simulink model "mb1k-v159"  (R2013a)
settings: Solver=VariableStepDiscrete  SolverMode=SingleTasking  StartTime=0.0  StopTime=10^4  FixedStep=1  MaxStep=1  RelTol=1e-3
undefined symbols (not defined in the file): pfb_coeff_gen_calc, sfp
libraries: xbsIndex_r4 x25251, casper_library_misc x140, casper_library_delays x74, simulink x34, dspsigops x24, xbsReplacements_r4 x20, fast_library x16, xps_library x13, casper_library_sources x2
(part 3 of 256)

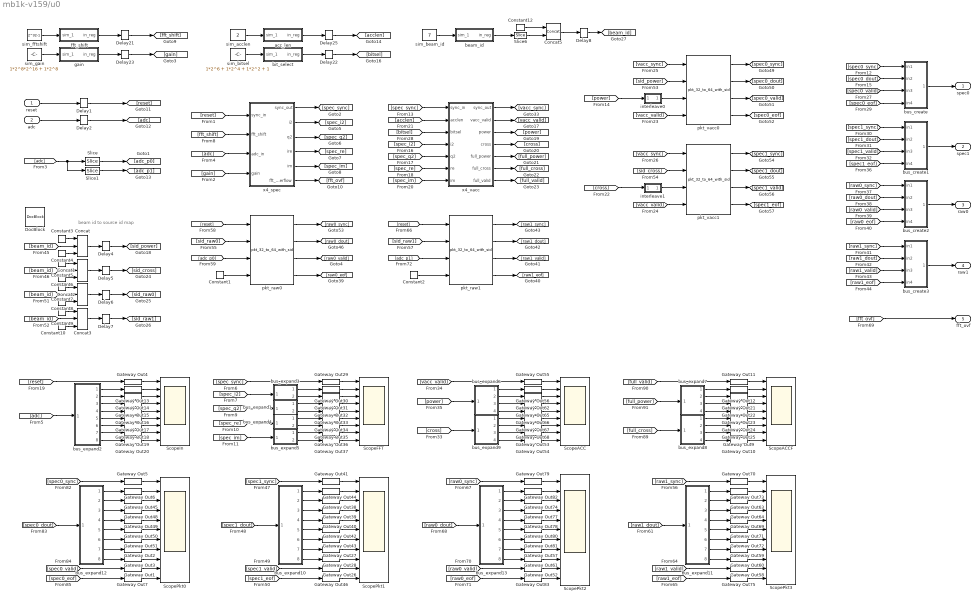
== system mb1k-v159/u0 ==
  note: beam id to source id map
  reset : Inport
  adc : Inport  Port=2
  Concat{,1..3} (4 alike) : xbsIndex_r4/Concat  num_inputs=3  dbl_ovrd=off  block_type=concat
  Concat5 : xbsIndex_r4/Concat  num_inputs=2  dbl_ovrd=off  block_type=concat
  Constant{1..2,6..7,9..10} (6 alike) : xbsIndex_r4/Constant  const=1  gui_display_data_type=Boolean  arith_type=Boolean  n_bits=16  bin_pt=14  preci_type=Single  exp_width=8  frac_width=24  explicit_period=on  period=1  equ=P=C  opselect=C  inp2=PCIN>>17  opr=+  inp1=P  carry=CIN  dbl_ovrd=off  block_type=constant
  Constant12 : xbsIndex_r4/Constant  const=1  gui_display_data_type=Fixed-point  arith_type=Unsigned  n_bits=1  bin_pt=0  preci_type=Single  exp_width=8  frac_width=24  explicit_period=on  period=1  equ=P=C  opselect=C  inp2=PCIN>>17  opr=+  inp1=P  carry=CIN  dbl_ovrd=off  block_type=constant
  Constant{3..5,8} (4 alike) : xbsIndex_r4/Constant  const=0  gui_display_data_type=Boolean  arith_type=Boolean  n_bits=16  bin_pt=14  preci_type=Single  exp_width=8  frac_width=24  explicit_period=on  period=1  equ=P=C  opselect=C  inp2=PCIN>>17  opr=+  inp1=P  carry=CIN  dbl_ovrd=off  block_type=constant
  Delay{1..2,4..8,21..23,25} (11 alike) : xbsIndex_r4/Delay  rst=off  infoeditControl=Selection of Reset will increase slice count due to use of real FFs and instead of SRLs  en=off  latency=1  dbl_ovrd=off  reg_retiming=off  block_type=delay
  DocBlock : simulink/Model-Wide Utilities/DocBlock  DocumentType=Text
      content=|
      | Highest byte of 64-bit little endian packet serial number to represent source id:
      |
      | bit 7:    0 - power spectra, 1 - pure ADC sample
      | bit 6:    0 - single beam, 1 - multi-beam
      | bit 5-1:  5 bits, 32 values for beam id.
      |           multi-beam: 1-19 (I reserved number 0)
      |           single-beam: TBD
      | bit 0:    ADC data: polarization
      |           spectra: 0 - power term, 1 - cross term
  Gateway Out{1..7,9..46,48..75,77..83} (80 alike) : xbsIndex_r4/Gateway Out  inherit_from_input=off  hdl_port=on  timing_constraint=None  locs_specified=off  LOCs={}  UseAsDAC=off  DACChannel='1'  block_type=gatewayout
  ScopeACC : Scope  NumInputPorts=9
      settings: YMin=-5~-5~-5~-5~-5~-5~-5~-5~-5  YMax=5~5~5~5~5~5~5~5~5  SaveName=u0ScopeACC
  ScopeACCF : Scope  NumInputPorts=9
      settings: YMin=-5~-5~-5~-5~-5~-5~-5~-5~-5  YMax=5~5~5~5~5~5~5~5~5  SaveName=u0ScopeACCF
  ScopeFFT : Scope  NumInputPorts=9
      settings: YMin=-5~-5~-5~-5~-5~-5~-5~-5~-5  YMax=5~5~5~5~5~5~5~5~5  SaveName=u0ScopeFFT
  ScopeIn : Scope  NumInputPorts=9
      settings: YMin=-5~-0.1~-5~-5~-5~-5~-5~-5~-5  YMax=5~0.1~5~5~5~5~5~5~5  SaveName=u0ScopeIn
  ScopePkt{0..3} (4 alike) : Scope  NumInputPorts=11
      ScopePkt0 settings: YMin=-5~-5~-5~-5~-5~-5~-5~-5~-5~-5~-5  YMax=5~5~5~5~5~5~5~5~5~5~5  SaveName=u0ScopePkt0
      ScopePkt1 settings: YMin=-5~-5~-5~-5~-5~-5~-5~-5~-5~-5~-5  YMax=5~5~5~5~5~5~5~5~5~5~5  SaveName=u0ScopePkt1
      ScopePkt2 settings: YMin=-5~-5~-5~-5~-5~-5~-5~-5~-5~-5~-5  YMax=5~5~5~5~5~5~5~5~5~5~5  SaveName=u0ScopePkt2
      ScopePkt3 settings: YMin=-5~-5~-5~-5~-5~-5~-5~-5~-5~-5~-5  YMax=5~5~5~5~5~5~5~5~5~5~5  SaveName=u0ScopePkt3
  Slice : xbsIndex_r4/Slice  nbits=32  boolean_output=off  mode=Upper Bit Location + Width  bit1=0  base1=MSB of Input  bit0=0  base0=LSB of Input  dbl_ovrd=off  block_type=slice
  Slice1 : xbsIndex_r4/Slice  nbits=32  boolean_output=off  mode=Lower Bit Location + Width  bit1=0  base1=MSB of Input  bit0=0  base0=LSB of Input  dbl_ovrd=off  block_type=slice
  Slice6 : xbsIndex_r4/Slice  nbits=5  boolean_output=off  mode=Lower Bit Location + Width  bit1=0  base1=MSB of Input  bit0=0  base0=LSB of Input  dbl_ovrd=off  block_type=slice
  acc_len : Subsystem  Tag=xps:sw_reg  AncestorBlock=xps_library/software register  mask(swreg){io_dir=From Processor, io_delay=0, sample_period=1, names=reg, bitwidths=20, bin_pts=0, arith_types=0, sim_port=on, show_format=off}
      mask prompts: io_dir "I/O direction"; io_delay "I/O delay"; sample_period "Sample period"; names "Bitfield names [msb...lsb]"; bitwidths "Bitfield widths"; bin_pts "Bitfield binary pts"; arith_types "Bitfield types, ufix=0, fix=1, bool=2"; sim_port "Provide sim input/output?"; show_format "Print format string?"
      mask help: A 32-bit software-accessible register. Can be divided into bitfields of varying widths and types using the fields in the block mask.
      mask init:
      | swreg_init(gcb)
      settings: AttributesFormatString=1input
      mask TypeOptions io_dir: To Processor | From Processor
      mask Callback io_dir:
      | mask_enables = get_param(gcb, 'MaskEnables');
      | mask_names = get_param(gcb, 'MaskNames');
      | if strcmp(get_param(gcb, 'io_dir'), 'To Processor'),
      |     mask_enables{ismember(mask_names, 'sample_period')} = 'off';
      | else
      |     mask_enables{ismember(mask_names, 'sample_period')} = 'on';
      | end
      | set_param(gcb, 'MaskEnables', mask_enables);
  beam_id : Subsystem  Tag=xps:sw_reg  AncestorBlock=xps_library/software register  mask(swreg){io_dir=From Processor, io_delay=0, sample_period=1, names=reg, bitwidths=5, bin_pts=0, arith_types=0, sim_port=on, show_format=off}
      mask prompts: io_dir "I/O direction"; io_delay "I/O delay"; sample_period "Sample period"; names "Bitfield names [msb...lsb]"; bitwidths "Bitfield widths"; bin_pts "Bitfield binary pts"; arith_types "Bitfield types, ufix=0, fix=1, bool=2"; sim_port "Provide sim input/output?"; show_format "Print format string?"
      mask help: A 32-bit software-accessible register. Can be divided into bitfields of varying widths and types using the fields in the block mask.
      mask init:
      | swreg_init(gcb)
      settings: AttributesFormatString=1input
      mask TypeOptions io_dir: To Processor | From Processor
      mask Callback io_dir:
      | mask_enables = get_param(gcb, 'MaskEnables');
      | mask_names = get_param(gcb, 'MaskNames');
      | if strcmp(get_param(gcb, 'io_dir'), 'To Processor'),
      |     mask_enables{ismember(mask_names, 'sample_period')} = 'off';
      | else
      |     mask_enables{ismember(mask_names, 'sample_period')} = 'on';
      | end
      | set_param(gcb, 'MaskEnables', mask_enables);
  bit_select : Subsystem  Tag=xps:sw_reg  AncestorBlock=xps_library/software register  mask(swreg){io_dir=From Processor, io_delay=0, sample_period=1, names=reg, bitwidths=8, bin_pts=0, arith_types=0, sim_port=on, show_format=off}
      mask prompts: io_dir "I/O direction"; io_delay "I/O delay"; sample_period "Sample period"; names "Bitfield names [msb...lsb]"; bitwidths "Bitfield widths"; bin_pts "Bitfield binary pts"; arith_types "Bitfield types, ufix=0, fix=1, bool=2"; sim_port "Provide sim input/output?"; show_format "Print format string?"
      mask help: A 32-bit software-accessible register. Can be divided into bitfields of varying widths and types using the fields in the block mask.
      mask init:
      | swreg_init(gcb)
      settings: AttributesFormatString=1input
      mask TypeOptions io_dir: To Processor | From Processor
      mask Callback io_dir:
      | mask_enables = get_param(gcb, 'MaskEnables');
      | mask_names = get_param(gcb, 'MaskNames');
      | if strcmp(get_param(gcb, 'io_dir'), 'To Processor'),
      |     mask_enables{ismember(mask_names, 'sample_period')} = 'off';
      | else
      |     mask_enables{ismember(mask_names, 'sample_period')} = 'on';
      | end
      | set_param(gcb, 'MaskEnables', mask_enables);
  bus_create : Subsystem  AncestorBlock=casper_library_flow_control/bus_create  mask(bus_create){inputNum=4}
      mask prompts: inputNum "Number of inputs:"
      mask init:
      | bus_create_init(gcb,...
      |     'inputNum', inputNum);
  bus_create1 : Subsystem  AncestorBlock=casper_library_flow_control/bus_create  mask(bus_create){inputNum=4}
      mask prompts: inputNum "Number of inputs:"
      mask init:
      | bus_create_init(gcb,...
      |     'inputNum', inputNum);
  bus_create2 : Subsystem  AncestorBlock=casper_library_flow_control/bus_create  mask(bus_create){inputNum=4}
      mask prompts: inputNum "Number of inputs:"
      mask init:
      | bus_create_init(gcb,...
      |     'inputNum', inputNum);
  bus_create3 : Subsystem  AncestorBlock=casper_library_flow_control/bus_create  mask(bus_create){inputNum=4}
      mask prompts: inputNum "Number of inputs:"
      mask init:
      | bus_create_init(gcb,...
      |     'inputNum', inputNum);
  bus_expand1 : Subsystem  AncestorBlock=casper_library_flow_control/bus_expand  mask(bus_expand){mode=divisions of equal size, outputNum=2, outputWidth=36, outputBinaryPt=34, outputArithmeticType=0, show_format=off, outputToWorkspace=off, variablePrefix=out, outputToModelAsWell=on}
      mask prompts: outputNum "Number of outputs:"; outputWidth "Output width:"; outputBinaryPt "Output binary point position:"; outputArithmeticType "Output arithmetic type (ufix=0, fix=1, bool=2):"; show_format "Print format string?"; outputToWorkspace "Output to workspace?"; variablePrefix "Variable name prefix:"; outputToModelAsWell "Output to model as well?"
      mask help:
      | Expands a 'bus' made using a bus_create or Xilinx
      | concat block.
      | The input will be divided into the specified number
      | of outputs with the specified arithmetic types.
      | Divisions are made from most significant bit.
      mask init: same as mb1k-v159/bus_expand
      settings: AttributesFormatString=2 outputs
      mask TypeOptions mode: divisions of equal size | divisions of arbitrary size
      mask Callback mode: bus_expand_callback();
      mask Callback outputWidth: bus_expand_callback();
      mask Callback outputToWorkspace: bus_expand_callback();
  bus_expand10 : Subsystem  AncestorBlock=casper_library_flow_control/bus_expand  mask(bus_expand){mode=divisions of equal size, outputNum=8, outputWidth=8, outputBinaryPt=0, outputArithmeticType=0, show_format=off, outputToWorkspace=off, variablePrefix=out, outputToModelAsWell=on}
      mask prompts: outputNum "Number of outputs:"; outputWidth "Output width:"; outputBinaryPt "Output binary point position:"; outputArithmeticType "Output arithmetic type (ufix=0, fix=1, bool=2):"; show_format "Print format string?"; outputToWorkspace "Output to workspace?"; variablePrefix "Variable name prefix:"; outputToModelAsWell "Output to model as well?"
      mask help:
      | Expands a 'bus' made using a bus_create or Xilinx
      | concat block.
      | The input will be divided into the specified number
      | of outputs with the specified arithmetic types.
      | Divisions are made from most significant bit.
      mask init: same as mb1k-v159/bus_expand
      settings: AttributesFormatString=8 outputs
      mask TypeOptions mode: divisions of equal size | divisions of arbitrary size
      mask Callback mode: bus_expand_callback();
      mask Callback outputWidth: bus_expand_callback();
      mask Callback outputToWorkspace: bus_expand_callback();
  bus_expand11 : Subsystem  AncestorBlock=casper_library_flow_control/bus_expand  mask(bus_expand){mode=divisions of equal size, outputNum=8, outputWidth=8, outputBinaryPt=0, outputArithmeticType=0, show_format=off, outputToWorkspace=off, variablePrefix=out, outputToModelAsWell=on}
      mask prompts: outputNum "Number of outputs:"; outputWidth "Output width:"; outputBinaryPt "Output binary point position:"; outputArithmeticType "Output arithmetic type (ufix=0, fix=1, bool=2):"; show_format "Print format string?"; outputToWorkspace "Output to workspace?"; variablePrefix "Variable name prefix:"; outputToModelAsWell "Output to model as well?"
      mask help:
      | Expands a 'bus' made using a bus_create or Xilinx
      | concat block.
      | The input will be divided into the specified number
      | of outputs with the specified arithmetic types.
      | Divisions are made from most significant bit.
      mask init: same as mb1k-v159/bus_expand
      settings: AttributesFormatString=8 outputs
      mask TypeOptions mode: divisions of equal size | divisions of arbitrary size
      mask Callback mode: bus_expand_callback();
      mask Callback outputWidth: bus_expand_callback();
      mask Callback outputToWorkspace: bus_expand_callback();
  bus_expand12 : Subsystem  AncestorBlock=casper_library_flow_control/bus_expand  mask(bus_expand){mode=divisions of equal size, outputNum=8, outputWidth=8, outputBinaryPt=0, outputArithmeticType=0, show_format=off, outputToWorkspace=off, variablePrefix=out, outputToModelAsWell=on}
      mask prompts: outputNum "Number of outputs:"; outputWidth "Output width:"; outputBinaryPt "Output binary point position:"; outputArithmeticType "Output arithmetic type (ufix=0, fix=1, bool=2):"; show_format "Print format string?"; outputToWorkspace "Output to workspace?"; variablePrefix "Variable name prefix:"; outputToModelAsWell "Output to model as well?"
      mask help:
      | Expands a 'bus' made using a bus_create or Xilinx
      | concat block.
      | The input will be divided into the specified number
      | of outputs with the specified arithmetic types.
      | Divisions are made from most significant bit.
      mask init: same as mb1k-v159/bus_expand
      settings: AttributesFormatString=8 outputs
      mask TypeOptions mode: divisions of equal size | divisions of arbitrary size
      mask Callback mode: bus_expand_callback();
      mask Callback outputWidth: bus_expand_callback();
      mask Callback outputToWorkspace: bus_expand_callback();
  bus_expand13 : Subsystem  AncestorBlock=casper_library_flow_control/bus_expand  mask(bus_expand){mode=divisions of equal size, outputNum=8, outputWidth=8, outputBinaryPt=0, outputArithmeticType=0, show_format=off, outputToWorkspace=off, variablePrefix=out, outputToModelAsWell=on}
      mask prompts: outputNum "Number of outputs:"; outputWidth "Output width:"; outputBinaryPt "Output binary point position:"; outputArithmeticType "Output arithmetic type (ufix=0, fix=1, bool=2):"; show_format "Print format string?"; outputToWorkspace "Output to workspace?"; variablePrefix "Variable name prefix:"; outputToModelAsWell "Output to model as well?"
      mask help:
      | Expands a 'bus' made using a bus_create or Xilinx
      | concat block.
      | The input will be divided into the specified number
      | of outputs with the specified arithmetic types.
      | Divisions are made from most significant bit.
      mask init: same as mb1k-v159/bus_expand
      settings: AttributesFormatString=8 outputs
      mask TypeOptions mode: divisions of equal size | divisions of arbitrary size
      mask Callback mode: bus_expand_callback();
      mask Callback outputWidth: bus_expand_callback();
      mask Callback outputToWorkspace: bus_expand_callback();
  bus_expand2 : Subsystem  AncestorBlock=casper_library_flow_control/bus_expand  mask(bus_expand){mode=divisions of equal size, outputNum=8, outputWidth=8, outputBinaryPt=7, outputArithmeticType=1, show_format=off, outputToWorkspace=off, variablePrefix=out, outputToModelAsWell=on}
      mask prompts: outputNum "Number of outputs:"; outputWidth "Output width:"; outputBinaryPt "Output binary point position:"; outputArithmeticType "Output arithmetic type (ufix=0, fix=1, bool=2):"; show_format "Print format string?"; outputToWorkspace "Output to workspace?"; variablePrefix "Variable name prefix:"; outputToModelAsWell "Output to model as well?"
      mask help:
      | Expands a 'bus' made using a bus_create or Xilinx
      | concat block.
      | The input will be divided into the specified number
      | of outputs with the specified arithmetic types.
      | Divisions are made from most significant bit.
      mask init: same as mb1k-v159/bus_expand
      settings: AttributesFormatString=8 outputs
      mask TypeOptions mode: divisions of equal size | divisions of arbitrary size
      mask Callback mode: bus_expand_callback();
      mask Callback outputWidth: bus_expand_callback();
      mask Callback outputToWorkspace: bus_expand_callback();
  bus_expand3 : Subsystem  AncestorBlock=casper_library_flow_control/bus_expand  mask(bus_expand){mode=divisions of equal size, outputNum=2, outputWidth=36, outputBinaryPt=34, outputArithmeticType=0, show_format=off, outputToWorkspace=off, variablePrefix=out, outputToModelAsWell=on}
      mask prompts: outputNum "Number of outputs:"; outputWidth "Output width:"; outputBinaryPt "Output binary point position:"; outputArithmeticType "Output arithmetic type (ufix=0, fix=1, bool=2):"; show_format "Print format string?"; outputToWorkspace "Output to workspace?"; variablePrefix "Variable name prefix:"; outputToModelAsWell "Output to model as well?"
      mask help:
      | Expands a 'bus' made using a bus_create or Xilinx
      | concat block.
      | The input will be divided into the specified number
      | of outputs with the specified arithmetic types.
      | Divisions are made from most significant bit.
      mask init: same as mb1k-v159/bus_expand
      settings: AttributesFormatString=2 outputs
      mask TypeOptions mode: divisions of equal size | divisions of arbitrary size
      mask Callback mode: bus_expand_callback();
      mask Callback outputWidth: bus_expand_callback();
      mask Callback outputToWorkspace: bus_expand_callback();
  bus_expand4 : Subsystem  AncestorBlock=casper_library_flow_control/bus_expand  mask(bus_expand){mode=divisions of equal size, outputNum=2, outputWidth=36, outputBinaryPt=34, outputArithmeticType=1, show_format=off, outputToWorkspace=off, variablePrefix=out, outputToModelAsWell=on}
      mask prompts: outputNum "Number of outputs:"; outputWidth "Output width:"; outputBinaryPt "Output binary point position:"; outputArithmeticType "Output arithmetic type (ufix=0, fix=1, bool=2):"; show_format "Print format string?"; outputToWorkspace "Output to workspace?"; variablePrefix "Variable name prefix:"; outputToModelAsWell "Output to model as well?"
      mask help:
      | Expands a 'bus' made using a bus_create or Xilinx
      | concat block.
      | The input will be divided into the specified number
      | of outputs with the specified arithmetic types.
      | Divisions are made from most significant bit.
      mask init: same as mb1k-v159/bus_expand
      settings: AttributesFormatString=2 outputs
      mask TypeOptions mode: divisions of equal size | divisions of arbitrary size
      mask Callback mode: bus_expand_callback();
      mask Callback outputWidth: bus_expand_callback();
      mask Callback outputToWorkspace: bus_expand_callback();
  bus_expand5 : Subsystem  AncestorBlock=casper_library_flow_control/bus_expand  mask(bus_expand){mode=divisions of equal size, outputNum=2, outputWidth=36, outputBinaryPt=34, outputArithmeticType=1, show_format=off, outputToWorkspace=off, variablePrefix=out, outputToModelAsWell=on}
      mask prompts: outputNum "Number of outputs:"; outputWidth "Output width:"; outputBinaryPt "Output binary point position:"; outputArithmeticType "Output arithmetic type (ufix=0, fix=1, bool=2):"; show_format "Print format string?"; outputToWorkspace "Output to workspace?"; variablePrefix "Variable name prefix:"; outputToModelAsWell "Output to model as well?"
      mask help:
      | Expands a 'bus' made using a bus_create or Xilinx
      | concat block.
      | The input will be divided into the specified number
      | of outputs with the specified arithmetic types.
      | Divisions are made from most significant bit.
      mask init: same as mb1k-v159/bus_expand
      settings: AttributesFormatString=2 outputs
      mask TypeOptions mode: divisions of equal size | divisions of arbitrary size
      mask Callback mode: bus_expand_callback();
      mask Callback outputWidth: bus_expand_callback();
      mask Callback outputToWorkspace: bus_expand_callback();
  bus_expand6 : Subsystem  AncestorBlock=casper_library_flow_control/bus_expand  mask(bus_expand){mode=divisions of equal size, outputNum=4, outputWidth=8, outputBinaryPt=0, outputArithmeticType=0, show_format=off, outputToWorkspace=off, variablePrefix=out, outputToModelAsWell=on}
      mask prompts: outputNum "Number of outputs:"; outputWidth "Output width:"; outputBinaryPt "Output binary point position:"; outputArithmeticType "Output arithmetic type (ufix=0, fix=1, bool=2):"; show_format "Print format string?"; outputToWorkspace "Output to workspace?"; variablePrefix "Variable name prefix:"; outputToModelAsWell "Output to model as well?"
      mask help:
      | Expands a 'bus' made using a bus_create or Xilinx
      | concat block.
      | The input will be divided into the specified number
      | of outputs with the specified arithmetic types.
      | Divisions are made from most significant bit.
      mask init: same as mb1k-v159/bus_expand
      settings: AttributesFormatString=4 outputs
      mask TypeOptions mode: divisions of equal size | divisions of arbitrary size
      mask Callback mode: bus_expand_callback();
      mask Callback outputWidth: bus_expand_callback();
      mask Callback outputToWorkspace: bus_expand_callback();
  bus_expand7 : Subsystem  AncestorBlock=casper_library_flow_control/bus_expand  mask(bus_expand){mode=divisions of equal size, outputNum=4, outputWidth=32, outputBinaryPt=0, outputArithmeticType=0, show_format=off, outputToWorkspace=off, variablePrefix=out, outputToModelAsWell=on}
      mask prompts: outputNum "Number of outputs:"; outputWidth "Output width:"; outputBinaryPt "Output binary point position:"; outputArithmeticType "Output arithmetic type (ufix=0, fix=1, bool=2):"; show_format "Print format string?"; outputToWorkspace "Output to workspace?"; variablePrefix "Variable name prefix:"; outputToModelAsWell "Output to model as well?"
      mask help:
      | Expands a 'bus' made using a bus_create or Xilinx
      | concat block.
      | The input will be divided into the specified number
      | of outputs with the specified arithmetic types.
      | Divisions are made from most significant bit.
      mask init: same as mb1k-v159/bus_expand
      settings: AttributesFormatString=4 outputs
      mask TypeOptions mode: divisions of equal size | divisions of arbitrary size
      mask Callback mode: bus_expand_callback();
      mask Callback outputWidth: bus_expand_callback();
      mask Callback outputToWorkspace: bus_expand_callback();
  bus_expand8 : Subsystem  AncestorBlock=casper_library_flow_control/bus_expand  mask(bus_expand){mode=divisions of equal size, outputNum=4, outputWidth=32, outputBinaryPt=0, outputArithmeticType=1, show_format=off, outputToWorkspace=off, variablePrefix=out, outputToModelAsWell=on}
      mask prompts: outputNum "Number of outputs:"; outputWidth "Output width:"; outputBinaryPt "Output binary point position:"; outputArithmeticType "Output arithmetic type (ufix=0, fix=1, bool=2):"; show_format "Print format string?"; outputToWorkspace "Output to workspace?"; variablePrefix "Variable name prefix:"; outputToModelAsWell "Output to model as well?"
      mask help:
      | Expands a 'bus' made using a bus_create or Xilinx
      | concat block.
      | The input will be divided into the specified number
      | of outputs with the specified arithmetic types.
      | Divisions are made from most significant bit.
      mask init: same as mb1k-v159/bus_expand
      settings: AttributesFormatString=4 outputs
      mask TypeOptions mode: divisions of equal size | divisions of arbitrary size
      mask Callback mode: bus_expand_callback();
      mask Callback outputWidth: bus_expand_callback();
      mask Callback outputToWorkspace: bus_expand_callback();
  bus_expand9 : Subsystem  AncestorBlock=casper_library_flow_control/bus_expand  mask(bus_expand){mode=divisions of equal size, outputNum=4, outputWidth=8, outputBinaryPt=0, outputArithmeticType=1, show_format=off, outputToWorkspace=off, variablePrefix=out, outputToModelAsWell=on}
      mask prompts: outputNum "Number of outputs:"; outputWidth "Output width:"; outputBinaryPt "Output binary point position:"; outputArithmeticType "Output arithmetic type (ufix=0, fix=1, bool=2):"; show_format "Print format string?"; outputToWorkspace "Output to workspace?"; variablePrefix "Variable name prefix:"; outputToModelAsWell "Output to model as well?"
      mask help:
      | Expands a 'bus' made using a bus_create or Xilinx
      | concat block.
      | The input will be divided into the specified number
      | of outputs with the specified arithmetic types.
      | Divisions are made from most significant bit.
      mask init: same as mb1k-v159/bus_expand
      settings: AttributesFormatString=4 outputs
      mask TypeOptions mode: divisions of equal size | divisions of arbitrary size
      mask Callback mode: bus_expand_callback();
      mask Callback outputWidth: bus_expand_callback();
      mask Callback outputToWorkspace: bus_expand_callback();
  fft_shift : Subsystem  Tag=xps:sw_reg  AncestorBlock=xps_library/software register  mask(swreg){io_dir=From Processor, io_delay=0, sample_period=1, names=reg, bitwidths=16, bin_pts=0, arith_types=0, sim_port=on, show_format=off}
      mask prompts: io_dir "I/O direction"; io_delay "I/O delay"; sample_period "Sample period"; names "Bitfield names [msb...lsb]"; bitwidths "Bitfield widths"; bin_pts "Bitfield binary pts"; arith_types "Bitfield types, ufix=0, fix=1, bool=2"; sim_port "Provide sim input/output?"; show_format "Print format string?"
      mask help: A 32-bit software-accessible register. Can be divided into bitfields of varying widths and types using the fields in the block mask.
      mask init:
      | swreg_init(gcb)
      settings: AttributesFormatString=1input
      mask TypeOptions io_dir: To Processor | From Processor
      mask Callback io_dir:
      | mask_enables = get_param(gcb, 'MaskEnables');
      | mask_names = get_param(gcb, 'MaskNames');
      | if strcmp(get_param(gcb, 'io_dir'), 'To Processor'),
      |     mask_enables{ismember(mask_names, 'sample_period')} = 'off';
      | else
      |     mask_enables{ismember(mask_names, 'sample_period')} = 'on';
      | end
      | set_param(gcb, 'MaskEnables', mask_enables);
  gain : Subsystem  Tag=xps:sw_reg  AncestorBlock=xps_library/software register  mask(swreg){io_dir=From Processor, io_delay=0, sample_period=1, names=reg, bitwidths=32, bin_pts=0, arith_types=0, sim_port=on, show_format=off}
      mask prompts: io_dir "I/O direction"; io_delay "I/O delay"; sample_period "Sample period"; names "Bitfield names [msb...lsb]"; bitwidths "Bitfield widths"; bin_pts "Bitfield binary pts"; arith_types "Bitfield types, ufix=0, fix=1, bool=2"; sim_port "Provide sim input/output?"; show_format "Print format string?"
      mask help: A 32-bit software-accessible register. Can be divided into bitfields of varying widths and types using the fields in the block mask.
      mask init:
      | swreg_init(gcb)
      settings: AttributesFormatString=1input
      mask TypeOptions io_dir: To Processor | From Processor
      mask Callback io_dir:
      | mask_enables = get_param(gcb, 'MaskEnables');
      | mask_names = get_param(gcb, 'MaskNames');
      | if strcmp(get_param(gcb, 'io_dir'), 'To Processor'),
      |     mask_enables{ismember(mask_names, 'sample_period')} = 'off';
      | else
      |     mask_enables{ismember(mask_names, 'sample_period')} = 'on';
      | end
      | set_param(gcb, 'MaskEnables', mask_enables);
  interleave0 : Subsystem
  interleave1 : Subsystem
  pkt_raw0 : fast_library/pkt_32_to_64_with_sid  nbits=10
      settings: AttributesFormatString=packet length: 4104 bytes
  pkt_raw1 : fast_library/pkt_32_to_64_with_sid  nbits=10
      settings: AttributesFormatString=packet length: 4104 bytes
  pkt_vacc0 : fast_library/pkt_32_to_64_with_sid  nbits=9
      settings: AttributesFormatString=packet length: 2056 bytes
  pkt_vacc1 : fast_library/pkt_32_to_64_with_sid  nbits=9
      settings: AttributesFormatString=packet length: 2056 bytes
  sim_acclen : Constant  Value=2
  sim_beam_id : Constant  Value=7
  sim_bitsel : Constant  Value=1*2^6 + 1*2^4 + 1*2^2 + 1
  sim_fftshift : Constant  Value=2^32-1
  sim_gain : Constant  Value=1*2^8*2^16 + 1*2^8
  x4_spec : Subsystem  mask{FFTSize=11}
      mask prompts: FFTSize "Size of FFT: (2^? pts)"
  x4_vacc : Subsystem  mask{bin_bits=10}
      mask prompts: bin_bits "bin number: (2^?)"
  spec0 : Outport
  spec1 : Outport  Port=2
  raw0 : Outport  Port=3
  raw1 : Outport  Port=4
  fft_ovf : Outport  Port=5
  wires:
  fft_shift/1(in_reg) -> Delay21/1
  sim_fftshift/1 -> fft_shift/1(sim_1)
  Delay21/1 -> [fft_shift]
  [fft_shift] -> x4_spec/2(fft_shift)
  gain/1(in_reg) -> Delay23/1
  sim_gain/1 -> gain/1(sim_1)
  Delay23/1 -> [gain]
  [gain] -> x4_spec/4(gain)
  x4_spec/1(sync_out) -> [spec_sync]
  x4_spec/2(i2) -> [spec_i2]
  x4_spec/3(q2) -> [spec_q2]
  x4_spec/4(ire) -> [spec_re]
  x4_spec/5(im) -> [spec_im]
  x4_spec/6(fft_overflow) -> [fft_ovf]
  [spec_sync] -> x4_vacc/1(sync_in)
  [reset] -> x4_spec/1(sync_in)
  [adc] -> x4_spec/3(adc_in)
  acc_len/1(in_reg) -> Delay25/1
  sim_acclen/1 -> acc_len/1(sim_1)
  bit_select/1(in_reg) -> Delay22/1
  sim_bitsel/1 -> bit_select/1(sim_1)
  Delay22/1 -> [bitsel]
  Delay25/1 -> [acclen]
  [spec_i2] -> x4_vacc/4(i2)
  [acclen] -> x4_vacc/2(acclen)
  [spec_re] -> x4_vacc/6(re)
  [spec_q2] -> x4_vacc/5(q2)
  [bitsel] -> x4_vacc/3(bitsel)
  [spec_im] -> x4_vacc/7(im)
  x4_vacc/3(power) -> [power]
  x4_vacc/2(vacc_valid) -> [vacc_valid]
  x4_vacc/5(full_power) -> [full_power]
  x4_vacc/4(cross) -> [cross]
  x4_vacc/7(full_valid) -> [full_valid]
  x4_vacc/6(full_cross) -> [full_cross]
  [vacc_valid] -> pkt_vacc0/4
  [power] -> interleave0/1(in)
  [vacc_sync] -> pkt_vacc0/1
  x4_vacc/1(sync_out) -> [vacc_sync]
  pkt_vacc0/4 -> [spec0_eof]
  pkt_vacc0/3 -> [spec0_valid]
  pkt_vacc0/2 -> [spec0_dout]
  pkt_vacc0/1 -> [spec0_sync]
  [vacc_sync] -> pkt_vacc1/1
  [cross] -> interleave1/1(in)
  [vacc_valid] -> pkt_vacc1/4
  pkt_vacc1/4 -> [spec1_eof]
  pkt_vacc1/3 -> [spec1_valid]
  pkt_vacc1/2 -> [spec1_dout]
  pkt_vacc1/1 -> [spec1_sync]
  [reset] -> pkt_raw0/1
  Constant1/1 -> pkt_raw0/4
  pkt_raw0/1 -> [raw0_sync]
  [reset] -> pkt_raw1/1
  pkt_raw1/1 -> [raw1_sync]
  pkt_raw0/2 -> [raw0_dout]
  pkt_raw0/3 -> [raw0_valid]
  pkt_raw0/4 -> [raw0_eof]
  pkt_raw1/4 -> [raw1_eof]
  pkt_raw1/3 -> [raw1_valid]
  pkt_raw1/2 -> [raw1_dout]
  reset/1 -> Delay1/1
  adc/1 -> Delay2/1
  [reset] -> Gateway Out4/1
  Gateway Out13/1 -> ScopeIn/2
  Gateway Out14/1 -> ScopeIn/3
  Gateway Out15/1 -> ScopeIn/4
  Gateway Out16/1 -> ScopeIn/5
  Gateway Out17/1 -> ScopeIn/6
  Gateway Out18/1 -> ScopeIn/7
  Gateway Out19/1 -> ScopeIn/8
  Gateway Out20/1 -> ScopeIn/9
  Gateway Out4/1 -> ScopeIn/1
  [adc] -> bus_expand2/1(bus_in)
  [spec_sync] -> Gateway Out29/1
  [spec_i2] -> bus_expand3/1(bus_in)
  [spec_q2] -> bus_expand1/1(bus_in)
  [spec_re] -> bus_expand4/1(bus_in)
  [spec_im] -> bus_expand5/1(bus_in)
  Gateway Out55/1 -> ScopeACC/1
  Gateway Out56/1 -> ScopeACC/2
  Gateway Out54/1 -> ScopeACC/9
  Gateway Out68/1 -> ScopeACC/7
  Gateway Out66/1 -> ScopeACC/5
  Gateway Out65/1 -> ScopeACC/4
  Gateway Out67/1 -> ScopeACC/6
  Gateway Out53/1 -> ScopeACC/8
  Gateway Out62/1 -> ScopeACC/3
  [vacc_valid] -> Gateway Out55/1
  [power] -> bus_expand6/1(bus_in)
  [cross] -> bus_expand9/1(bus_in)
  Gateway Out2/1 -> ScopePkt0/8
  Gateway Out5/1 -> ScopePkt0/1
  Gateway Out6/1 -> ScopePkt0/2
  Gateway Out50/1 -> ScopePkt0/6
  Gateway Out45/1 -> ScopePkt0/3
  Gateway Out51/1 -> ScopePkt0/7
  Gateway Out3/1 -> ScopePkt0/9
  Gateway Out48/1 -> ScopePkt0/4
  Gateway Out49/1 -> ScopePkt0/5
  Gateway Out7/1 -> ScopePkt0/11
  Gateway Out1/1 -> ScopePkt0/10
  [spec0_sync] -> Gateway Out5/1
  [spec0_dout] -> bus_expand12/1(bus_in)
  bus_expand12/1(msb_out8) -> Gateway Out6/1
  bus_expand12/2(out7) -> Gateway Out45/1
  bus_expand12/3(out6) -> Gateway Out48/1
  bus_expand12/4(out5) -> Gateway Out49/1
  bus_expand12/5(out4) -> Gateway Out50/1
  bus_expand12/6(out3) -> Gateway Out51/1
  bus_expand12/7(out2) -> Gateway Out2/1
  bus_expand12/8(lsb_out1) -> Gateway Out3/1
  [spec0_valid] -> Gateway Out1/1
  [spec0_eof] -> Gateway Out7/1
  bus_expand2/1(msb_out8) -> Gateway Out13/1
  bus_expand2/2(out7) -> Gateway Out14/1
  bus_expand2/3(out6) -> Gateway Out15/1
  bus_expand2/4(out5) -> Gateway Out16/1
  bus_expand2/5(out4) -> Gateway Out17/1
  bus_expand2/6(out3) -> Gateway Out18/1
  bus_expand2/7(out2) -> Gateway Out19/1
  bus_expand2/8(lsb_out1) -> Gateway Out20/1
  Gateway Out37/1 -> ScopeFFT/9
  Gateway Out29/1 -> ScopeFFT/1
  Gateway Out30/1 -> ScopeFFT/2
  Gateway Out31/1 -> ScopeFFT/3
  Gateway Out36/1 -> ScopeFFT/8
  Gateway Out32/1 -> ScopeFFT/4
  Gateway Out33/1 -> ScopeFFT/5
  Gateway Out34/1 -> ScopeFFT/6
  Gateway Out35/1 -> ScopeFFT/7
  bus_expand3/1(msb_out2) -> Gateway Out30/1
  bus_expand3/2(lsb_out1) -> Gateway Out31/1
  bus_expand1/1(msb_out2) -> Gateway Out32/1
  bus_expand1/2(lsb_out1) -> Gateway Out33/1
  bus_expand4/1(msb_out2) -> Gateway Out34/1
  bus_expand4/2(lsb_out1) -> Gateway Out35/1
  bus_expand5/1(msb_out2) -> Gateway Out36/1
  bus_expand5/2(lsb_out1) -> Gateway Out37/1
  bus_expand6/1(msb_out4) -> Gateway Out56/1
  bus_expand6/2(out3) -> Gateway Out62/1
  bus_expand6/3(out2) -> Gateway Out65/1
  bus_expand6/4(lsb_out1) -> Gateway Out66/1
  bus_expand9/1(msb_out4) -> Gateway Out67/1
  bus_expand9/2(out3) -> Gateway Out68/1
  bus_expand9/3(out2) -> Gateway Out53/1
  bus_expand9/4(lsb_out1) -> Gateway Out54/1
  Gateway Out11/1 -> ScopeACCF/1
  Gateway Out12/1 -> ScopeACCF/2
  Gateway Out10/1 -> ScopeACCF/9
  Gateway Out25/1 -> ScopeACCF/7
  Gateway Out23/1 -> ScopeACCF/5
  Gateway Out22/1 -> ScopeACCF/4
  Gateway Out24/1 -> ScopeACCF/6
  Gateway Out9/1 -> ScopeACCF/8
  Gateway Out21/1 -> ScopeACCF/3
  [full_valid] -> Gateway Out11/1
  [full_power] -> bus_expand7/1(bus_in)
  [full_cross] -> bus_expand8/1(bus_in)
  bus_expand7/1(msb_out4) -> Gateway Out12/1
  bus_expand7/2(out3) -> Gateway Out21/1
  bus_expand7/3(out2) -> Gateway Out22/1
  bus_expand7/4(lsb_out1) -> Gateway Out23/1
  bus_expand8/1(msb_out4) -> Gateway Out24/1
  bus_expand8/2(out3) -> Gateway Out25/1
  bus_expand8/3(out2) -> Gateway Out9/1
  bus_expand8/4(lsb_out1) -> Gateway Out10/1
  Gateway Out27/1 -> ScopePkt1/8
  Gateway Out41/1 -> ScopePkt1/1
  Gateway Out44/1 -> ScopePkt1/2
  Gateway Out42/1 -> ScopePkt1/6
  Gateway Out38/1 -> ScopePkt1/3
  Gateway Out43/1 -> ScopePkt1/7
  Gateway Out28/1 -> ScopePkt1/9
  Gateway Out39/1 -> ScopePkt1/4
  Gateway Out40/1 -> ScopePkt1/5
  Gateway Out46/1 -> ScopePkt1/11
  Gateway Out26/1 -> ScopePkt1/10
  [spec1_sync] -> Gateway Out41/1
  [spec1_dout] -> bus_expand10/1(bus_in)
  bus_expand10/1(msb_out8) -> Gateway Out44/1
  bus_expand10/2(out7) -> Gateway Out38/1
  bus_expand10/3(out6) -> Gateway Out39/1
  bus_expand10/4(out5) -> Gateway Out40/1
  bus_expand10/5(out4) -> Gateway Out42/1
  bus_expand10/6(out3) -> Gateway Out43/1
  bus_expand10/7(out2) -> Gateway Out27/1
  bus_expand10/8(lsb_out1) -> Gateway Out28/1
  [spec1_valid] -> Gateway Out26/1
  [spec1_eof] -> Gateway Out46/1
  Gateway Out57/1 -> ScopePkt2/8
  Gateway Out79/1 -> ScopePkt2/1
  Gateway Out82/1 -> ScopePkt2/2
  Gateway Out80/1 -> ScopePkt2/6
  Gateway Out74/1 -> ScopePkt2/3
  Gateway Out81/1 -> ScopePkt2/7
  Gateway Out61/1 -> ScopePkt2/9
  Gateway Out77/1 -> ScopePkt2/4
  Gateway Out78/1 -> ScopePkt2/5
  Gateway Out83/1 -> ScopePkt2/11
  Gateway Out52/1 -> ScopePkt2/10
  [raw0_sync] -> Gateway Out79/1
  [raw0_dout] -> bus_expand13/1(bus_in)
  bus_expand13/1(msb_out8) -> Gateway Out82/1
  bus_expand13/2(out7) -> Gateway Out74/1
  bus_expand13/3(out6) -> Gateway Out77/1
  bus_expand13/4(out5) -> Gateway Out78/1
  bus_expand13/5(out4) -> Gateway Out80/1
  bus_expand13/6(out3) -> Gateway Out81/1
  bus_expand13/7(out2) -> Gateway Out57/1
  bus_expand13/8(lsb_out1) -> Gateway Out61/1
  [raw0_valid] -> Gateway Out52/1
  [raw0_eof] -> Gateway Out83/1
  Gateway Out59/1 -> ScopePkt3/8
  Gateway Out70/1 -> ScopePkt3/1
  Gateway Out73/1 -> ScopePkt3/2
  Gateway Out71/1 -> ScopePkt3/6
  Gateway Out63/1 -> ScopePkt3/3
  Gateway Out72/1 -> ScopePkt3/7
  Gateway Out60/1 -> ScopePkt3/9
  Gateway Out64/1 -> ScopePkt3/4
  Gateway Out69/1 -> ScopePkt3/5
  Gateway Out75/1 -> ScopePkt3/11
  Gateway Out58/1 -> ScopePkt3/10
  [raw1_sync] -> Gateway Out70/1
  [raw1_dout] -> bus_expand11/1(bus_in)
  bus_expand11/1(msb_out8) -> Gateway Out73/1
  bus_expand11/2(out7) -> Gateway Out63/1
  bus_expand11/3(out6) -> Gateway Out64/1
  bus_expand11/4(out5) -> Gateway Out69/1
  bus_expand11/5(out4) -> Gateway Out71/1
  bus_expand11/6(out3) -> Gateway Out72/1
  bus_expand11/7(out2) -> Gateway Out59/1
  bus_expand11/8(lsb_out1) -> Gateway Out60/1
  [raw1_valid] -> Gateway Out58/1
  [raw1_eof] -> Gateway Out75/1
  Slice/1 -> [adc_p0]
  Slice1/1 -> [adc_p1]
  [adc_p0] -> pkt_raw0/3
  Delay1/1 -> [reset]
  Delay2/1 -> [adc]
  [adc] -> Slice1/1, Slice/1
  [spec0_valid] -> bus_create/3(in3)
  [spec0_sync] -> bus_create/1(in1)
  [spec0_dout] -> bus_create/2(in2)
  [spec0_eof] -> bus_create/4(in4)
  bus_create/1(bus_out) -> spec0/1
  [spec1_valid] -> bus_create1/3(in3)
  [spec1_sync] -> bus_create1/1(in1)
  [spec1_dout] -> bus_create1/2(in2)
  [spec1_eof] -> bus_create1/4(in4)
  bus_create1/1(bus_out) -> spec1/1
  [raw0_valid] -> bus_create2/3(in3)
  [raw0_sync] -> bus_create2/1(in1)
  [raw0_dout] -> bus_create2/2(in2)
  [raw0_eof] -> bus_create2/4(in4)
  bus_create2/1(bus_out) -> raw0/1
  [raw1_valid] -> bus_create3/3(in3)
  [raw1_sync] -> bus_create3/1(in1)
  [raw1_dout] -> bus_create3/2(in2)
  [raw1_eof] -> bus_create3/4(in4)
  bus_create3/1(bus_out) -> raw1/1
  [fft_ovf] -> fft_ovf/1
  interleave0/1(out) -> pkt_vacc0/3
  interleave1/1(out) -> pkt_vacc1/3
  Constant3/1 -> Concat/1
  Constant4/1 -> Concat/3
  [beam_id] -> Concat/2
  Concat/1 -> Delay4/1
  Delay4/1 -> [sid_power]
  Constant5/1 -> Concat1/1
  Constant6/1 -> Concat1/3
  [beam_id] -> Concat1/2
  Concat1/1 -> Delay5/1
  Delay5/1 -> [sid_cross]
  Constant7/1 -> Concat2/1
  Constant8/1 -> Concat2/3
  [beam_id] -> Concat2/2
  Concat2/1 -> Delay6/1
  Delay6/1 -> [sid_raw0]
  Constant9/1 -> Concat3/1
  Constant10/1 -> Concat3/3
  [beam_id] -> Concat3/2
  Concat3/1 -> Delay7/1
  Delay7/1 -> [sid_raw1]
  [sid_power] -> pkt_vacc0/2
  [sid_cross] -> pkt_vacc1/2
  [sid_raw0] -> pkt_raw0/2
  [adc_p1] -> pkt_raw1/3
  Constant2/1 -> pkt_raw1/4
  [sid_raw1] -> pkt_raw1/2
  sim_beam_id/1 -> beam_id/1(sim_1)
  beam_id/1(in_reg) -> Slice6/1
  Delay8/1 -> [beam_id]
  Slice6/1 -> Concat5/2
  Concat5/1 -> Delay8/1
  Constant12/1 -> Concat5/1
